simulink library "inp"  (Simulink 8.2)
settings: Solver=ode45  SolverMode=SingleTasking  StartTime=0.0  StopTime=10.0  RelTol=1e-3
undefined symbols (not defined in the file or in its folder): C_TICK_TIME_S, ENUM_FAULTSTATE_FAIL, ENUM_FAULTSTATE_INDETERMINATE, ENUM_FAULTSTATE_PASS, ENUM_KEYSWITCH_ON, FALSE, KINP_ABS1CANCommuOk_flg_ovrdflg, KINP_ABS1CANCommuOk_flg_ovrdval, KINP_ABS1CANPeriodTime_s, KINP_ABS1CANRolCntOk_flg_ovrdflg, KINP_ABS1CANRolCntOk_flg_ovrdval, KINP_ABS2CANChkSumOk_flg_ovrdflg, KINP_ABS2CANChkSumOk_flg_ovrdval, KINP_ABS2CANCommuOk_flg_ovrdflg, KINP_ABS2CANCommuOk_flg_ovrdval, KINP_ABS2CANPeriodTime_s, KINP_ABS2CANRolCntOk_flg_ovrdflg, KINP_ABS2CANRolCntOk_flg_ovrdval, KINP_ABS3CANCommuOk_flg_ovrdflg, KINP_ABS3CANCommuOk_flg_ovrdval, KINP_ABS4CANCommuOk_flg_ovrdflg, KINP_ABS4CANCommuOk_flg_ovrdval, KINP_ABSActive_flg_defval, KINP_ABSActive_flg_invalid, KINP_ABSActive_flg_ovrdflg, KINP_ABSActive_flg_ovrdval, KINP_ABSActive_flg_setvalid, KINP_ABSActive_flg_srvcflg, KINP_ABSActive_flg_srvcval, KINP_ABSCANAlive_flg_ovrdflg, KINP_ABSCANAlive_flg_ovrdval, KINP_ABSCANCommuOk_flg_ovrdflg, KINP_ABSCANCommuOk_flg_ovrdval, KINP_ABSCANValidOk_flg_ovrdflg, KINP_ABSCANValidOk_flg_ovrdval, KINP_ABSFault_flg_defval, KINP_ABSFault_flg_invalid, KINP_ABSFault_flg_ovrdflg, KINP_ABSFault_flg_ovrdval, KINP_ABSFault_flg_setvalid, KINP_ABSFault_flg_srvcflg, KINP_ABSFault_flg_srvcval, KINP_AC1CANCommuOk_flg_ovrdflg, KINP_AC1CANCommuOk_flg_ovrdval, KINP_ACCANAlive_flg_ovrdflg, KINP_ACCANAlive_flg_ovrdval, KINP_ACCANCommuOk_flg_ovrdflg, KINP_ACCANCommuOk_flg_ovrdval, KINP_ACCANValidOk_flg_ovrdflg, KINP_ACCANValidOk_flg_ovrdval, KINP_ACCCancelH_flg_defval, KINP_ACCCancelH_flg_invalid, KINP_ACCCancelH_flg_ovrdflg, KINP_ACCCancelH_flg_ovrdval, KINP_ACCCancelH_flg_setvalid, KINP_ACCCancelH_flg_srvcflg, KINP_ACCCancelH_flg_srvcval, KINP_AccelPedalPosn_pct_defval, KINP_AccelPedalPosn_pct_gain, KINP_AccelPedalPosn_pct_invalid ... (+1866)
libraries: inp x192, hcu_lib x34, simulink x33
(part 3 of 21)

== system inp/INP/ECM_signals ==
  BHAL_outputs : Inport  OutDataTypeStr=Bus: BHAL_outputs
  CANvalid_bus : Inport  Port=2
  AccelPedalPosn : Subsystem
      settings: RTWSystemCode=Inline
  Bus Selector1 : BusSelector  OutputSignals=VHAL_OBDWarnCycAchiv_flg
  Bus Selector{7,10,17,20,22} (5 alike) : BusSelector  OutputSignals=VINP_CANMsgValidECM1_flg
  Bus Selector11 : BusSelector  OutputSignals=VHAL_EngTqFriction_Nm,VHAL_EngTqFrictionInvalid_flg
  Bus Selector{12,24,26..27} (4 alike) : BusSelector  OutputSignals=VINP_CANMsgValidECM9_flg,VINP_CANMsgUpdatedECM9_flg
  Bus Selector13 : BusSelector  OutputSignals=VHAL_EngTqMax_Nm,VHAL_EngTqMaxInvalid_flg
  Bus Selector14 : BusSelector  OutputSignals=VHAL_EMSOpState_enum
  Bus Selector15 : BusSelector  OutputSignals=VHAL_EngTqMin_Nm,VHAL_EngTqMinInvalid_flg
  Bus Selector16 : BusSelector  OutputSignals=VINP_CANMsgValidECM2_flg
  Bus Selector18 : BusSelector  OutputSignals=VHAL_EngTqIndicated_Nm,VHAL_EngTqIndicatedInvalid_flg
  Bus Selector2 : BusSelector  OutputSignals=VINP_CANMsgValidECM2_flg
  Bus Selector21 : BusSelector  OutputSignals=VHAL_EngTqIndicatedCOR_Nm,VHAL_EngTqIndicatedCORInvalid_flg
  Bus Selector23 : BusSelector  OutputSignals=VHAL_EngTqUnCORFault_flg
  Bus Selector25 : BusSelector  OutputSignals=VHAL_EMSHybridOpState_enum
  Bus Selector28 : BusSelector  OutputSignals=VHAL_EngHoodOpen_flg
  Bus Selector3 : BusSelector  OutputSignals=VINP_CANMsgValidECM3_flg
  Bus Selector30 : BusSelector  OutputSignals=VINP_CANMsgValidECM2_flg
  Bus Selector4 : BusSelector  OutputSignals=VHAL_AccelPedalPosn_pct,VHAL_AccelPedalPosnInvalid_flg
  Bus Selector5 : BusSelector  OutputSignals=VINP_CANMsgValidECM3_flg
  Bus Selector6 : BusSelector  OutputSignals=VHAL_EffAccelPedalPosn_pct,VHAL_EffAccelPedalPosnInvalid_flg
  Bus Selector8 : BusSelector  OutputSignals=VHAL_OBDDriveCycAchiv_flg
  Bus Selector9 : BusSelector  OutputSignals=VHAL_EngSpd_erpm,VHAL_EngSpdInvalid_flg
  Constant : Constant  Value=TRUE
  Constant1 : Constant  Value=TRUE
  Data Store Read1 : DataStoreRead  DataStoreName=VDDML_ECM2CANCommuFault_AF_flg
  Data Store Read{10,13,25,27} (4 alike) : DataStoreRead  DataStoreName=VDDML_ECM9CANRolCntFault_AF_flg
  Data Store Read11 : DataStoreRead  DataStoreName=VDDML_CANEngTqIndSigFault_AF_flg
  Data Store Read12 : DataStoreRead  DataStoreName=VDDML_CANAPPSigFault_AF_flg
  Data Store Read{9,14,26,28} (4 alike) : DataStoreRead  DataStoreName=VDDML_ECM9CANCommuFault_AF_flg
  Data Store Read16 : DataStoreRead  DataStoreName=VDDML_CANEngSpdSigFault_AF_flg
  Data Store Read17 : DataStoreRead  DataStoreName=VDDML_ECM3CANCommuFault_AF_flg
  Data Store Read18 : DataStoreRead  DataStoreName=VDDML_CANEngTqFricSigFault_AF_flg
  Data Store Read19 : DataStoreRead  DataStoreName=VDDML_CANEngTqMaxSigFault_AF_flg
  Data Store Read2 : DataStoreRead  DataStoreName=VDDML_ECM2CANCommuFault_AF_flg
  Data Store Read20 : DataStoreRead  DataStoreName=VDDML_CANEngTqMinSigFault_AF_flg
  Data Store Read{5,8,21,23..24} (5 alike) : DataStoreRead  DataStoreName=VDDML_ECM1CANCommuFault_AF_flg
  Data Store Read29 : DataStoreRead  DataStoreName=VDDML_ECM2CANCommuFault_AF_flg
  Data Store Read30 : DataStoreRead  DataStoreName=VDDML_CANEngTqIndCORSigFault_AF_flg
  Data Store Read6 : DataStoreRead  DataStoreName=VDDML_CANEffAPPSigFault_AF_flg
  Data Store Read7 : DataStoreRead  DataStoreName=VDDML_ECM3CANCommuFault_AF_flg
  Data Type Conversion : DataTypeConversion  OutDataTypeStr=boolean
  Data Type Conversion1 : DataTypeConversion  OutDataTypeStr=boolean
  EMSHybridOpState : Subsystem
      settings: RTWSystemCode=Inline
  EMSOpState : Subsystem
      settings: RTWSystemCode=Inline
  EffAccelPedalPosn : Subsystem
      settings: RTWSystemCode=Inline
  EngHoodOpen : Subsystem
      settings: RTWSystemCode=Inline
  EngTqUnCORFault : Subsystem
      settings: RTWSystemCode=Inline
  EngineFrictionTorque : Subsystem
      settings: RTWSystemCode=Inline
  EngineIndicatedTorque : Subsystem
      settings: RTWSystemCode=Inline
  EngineIndicatedTorqueCorrected : Subsystem
      settings: RTWSystemCode=Inline
  EngineSpeed : Subsystem
      settings: RTWSystemCode=Inline
  EngineTorqueMax : Subsystem
      settings: RTWSystemCode=Inline
  EngineTorqueMin : Subsystem
      settings: RTWSystemCode=Inline
  Logical Operator{2..4,10,12,20,23,25} (8 alike) : Logic  Tag=struct('inputs','1','comment',struct('text','','placement',1),'logdata',struct('maxnumvals',inf,'plotchannels',[],'stepsize',0,'downsampling',1,'loggingmode',3,'channels',1),'operator','NOT','output',struct('class','default','type','Bool8','description','','address','','unit','','name','$S_$B'),'date',731430.46749816)  Operator=OR
  Logical Operator{5,8,11,13,15,17..19} (8 alike) : Logic  Tag=struct('inputs','1','comment',struct('text','','placement',1),'logdata',struct('maxnumvals',inf,'plotchannels',[],'stepsize',0,'downsampling',1,'loggingmode',3,'channels',1),'operator','NOT','output',struct('class','default','type','Bool8','description','','address','','unit','','name','$S_$B'),'date',731430.46749816)  Operator=NOT  Inputs=1
  Logical Operator14 : Logic  Tag=struct('inputs','1','comment',struct('text','','placement',1),'logdata',struct('maxnumvals',inf,'plotchannels',[],'stepsize',0,'downsampling',1,'loggingmode',3,'channels',1),'operator','NOT','output',struct('class','default','type','Bool8','description','','address','','unit','','name','$S_$B'),'date',731430.46749816)  Operator=OR  Inputs=3
  Logical Operator16 : Logic  Tag=struct('inputs','1','comment',struct('text','','placement',1),'logdata',struct('maxnumvals',inf,'plotchannels',[],'stepsize',0,'downsampling',1,'loggingmode',3,'channels',1),'operator','NOT','output',struct('class','default','type','Bool8','description','','address','','unit','','name','$S_$B'),'date',731430.46749816)  Operator=OR  Inputs=3
  Logical Operator{21..22,24,26} (4 alike) : Logic  Tag=struct('inputs','1','comment',struct('text','','placement',1),'logdata',struct('maxnumvals',inf,'plotchannels',[],'stepsize',0,'downsampling',1,'loggingmode',3,'channels',1),'operator','NOT','output',struct('class','default','type','Bool8','description','','address','','unit','','name','$S_$B'),'date',731430.46749816)
  Terminator{,1..3} (4 alike) : Terminator
  inputs_bus2 : BusCreator  Inputs=36
  ECM_signal_bus : Outport
  wires:
  CANvalid_bus/1 -> [CANvalid_bus]
  Logical Operator8/1 -> AccelPedalPosn/2(signal_valid_in)
  inputs_bus2/1 -> ECM_signal_bus/1
  Bus Selector4/1 -> AccelPedalPosn/1(signal_in)  "<VHAL_AccelPedalPosn_pct>"
  BHAL_outputs/1 -> [BHAL_outputs]
  [BHAL_outputs] -> Bus Selector4/1
  AccelPedalPosn/2(signal_valid_out) -> inputs_bus2/2  "VINP_AccelPedalPosnValid_flg_InHAL"
  AccelPedalPosn/1(signal_out) -> inputs_bus2/1  "VINP_AccelPedalPosn_pct_InHAL"
  Bus Selector4/2 -> Logical Operator8/1  "<VHAL_AccelPedalPosnInvalid_flg>"
  Data Store Read12/1 -> Logical Operator2/1
  [CANvalid_bus] -> Bus Selector3/1
  Bus Selector3/1 -> AccelPedalPosn/3(msg_valid)  "<VINP_CANMsgValidECM3_flg>"
  Logical Operator2/1 -> AccelPedalPosn/4(Active_fault_flg)
  Data Store Read17/1 -> Logical Operator2/2
  AccelPedalPosn/3(raw_signal_valid_out) -> inputs_bus2/3  "VINP_AccelPedalPosnSigOk_flg_InHAL"
  Logical Operator5/1 -> EffAccelPedalPosn/2(signal_valid_in)
  Bus Selector6/1 -> EffAccelPedalPosn/1(signal_in)  "<VHAL_EffAccelPedalPosn_pct>"
  [BHAL_outputs] -> Bus Selector6/1
  Bus Selector6/2 -> Logical Operator5/1  "<VHAL_EffAccelPedalPosnInvalid_flg>"
  Data Store Read6/1 -> Logical Operator4/1
  [CANvalid_bus] -> Bus Selector5/1
  Bus Selector5/1 -> EffAccelPedalPosn/3(msg_valid)  "<VINP_CANMsgValidECM3_flg>"
  Logical Operator4/1 -> EffAccelPedalPosn/4(Active_fault_flg)
  Data Store Read7/1 -> Logical Operator4/2
  EffAccelPedalPosn/1(signal_out) -> inputs_bus2/4  "VINP_EffAccelPedalPosn_pct_InHAL"
  EffAccelPedalPosn/2(signal_valid_out) -> inputs_bus2/5  "VINP_EffAccelPedalPosnValid_flg_InHAL"
  EffAccelPedalPosn/3(raw_signal_valid_out) -> inputs_bus2/6  "VINP_EffAccelPedalPosnSigOk_flg_InHAL"
  Logical Operator11/1 -> EngineSpeed/2(signal_valid_in)
  Bus Selector9/1 -> EngineSpeed/1(signal_in)  "<VHAL_EngSpd_erpm>"
  [BHAL_outputs] -> Bus Selector9/1
  Bus Selector9/2 -> Logical Operator11/1  "<VHAL_EngSpdInvalid_flg>"
  Data Store Read16/1 -> Logical Operator10/1
  [CANvalid_bus] -> Bus Selector7/1
  Bus Selector7/1 -> EngineSpeed/3(msg_valid)  "<VINP_CANMsgValidECM1_flg>"
  Logical Operator10/1 -> EngineSpeed/4(Active_fault_flg)
  Data Store Read8/1 -> Logical Operator10/2
  EngineSpeed/1(signal_out) -> inputs_bus2/7  "VINP_EngSpd_erpm_InHAL"
  EngineSpeed/2(signal_valid_out) -> inputs_bus2/8  "VINP_EngSpdValid_flg_InHAL"
  EngineSpeed/3(raw_signal_valid_out) -> inputs_bus2/9  "VINP_EngSpdSigOk_flg_InHAL"
  Logical Operator13/1 -> EngineFrictionTorque/2(signal_valid_in)
  Bus Selector11/1 -> EngineFrictionTorque/1(signal_in)  "<VHAL_EngTqFriction_Nm>"
  [BHAL_outputs] -> Bus Selector11/1
  Bus Selector11/2 -> Logical Operator13/1  "<VHAL_EngTqFrictionInvalid_flg>"
  Data Store Read18/1 -> Logical Operator12/1
  [CANvalid_bus] -> Bus Selector10/1
  Bus Selector10/1 -> EngineFrictionTorque/3(msg_valid)  "<VINP_CANMsgValidECM1_flg>"
  Logical Operator12/1 -> EngineFrictionTorque/4(Active_fault_flg)
  Data Store Read5/1 -> Logical Operator12/2
  EngineFrictionTorque/1(signal_out) -> inputs_bus2/10  "VINP_EngTqFriction_Nm_InHAL"
  EngineFrictionTorque/2(signal_valid_out) -> inputs_bus2/11  "VINP_EngTqFrictionValid_flg_InHAL"
  EngineFrictionTorque/3(raw_signal_valid_out) -> inputs_bus2/12  "VINP_EngTqFrictionSigOk_flg_InHAL"
  Logical Operator15/1 -> EngineTorqueMax/2(signal_valid_in)
  Bus Selector13/1 -> EngineTorqueMax/1(signal_in)  "<VHAL_EngTqMax_Nm>"
  [BHAL_outputs] -> Bus Selector13/1
  Bus Selector13/2 -> Logical Operator15/1  "<VHAL_EngTqMaxInvalid_flg>"
  [CANvalid_bus] -> Bus Selector12/1
  Bus Selector12/1 -> Logical Operator21/1  "<VINP_CANMsgValidECM9_flg>"
  Logical Operator14/1 -> EngineTorqueMax/4(Active_fault_flg)
  EngineTorqueMax/1(signal_out) -> inputs_bus2/21  "VINP_EngTqMax_Nm_InHAL"
  EngineTorqueMax/2(signal_valid_out) -> inputs_bus2/22  "VINP_EngTqMaxValid_flg_InHAL"
  EngineTorqueMax/3(raw_signal_valid_out) -> inputs_bus2/23  "VINP_EngTqMaxSigOk_flg_InHAL"
  Data Store Read19/1 -> Logical Operator14/1
  Data Store Read9/1 -> Logical Operator14/2
  Data Store Read10/1 -> Logical Operator14/3
  Logical Operator17/1 -> EngineTorqueMin/2(signal_valid_in)
  Bus Selector15/1 -> EngineTorqueMin/1(signal_in)  "<VHAL_EngTqMin_Nm>"
  [BHAL_outputs] -> Bus Selector15/1
  Bus Selector15/2 -> Logical Operator17/1  "<VHAL_EngTqMinInvalid_flg>"
  [CANvalid_bus] -> Bus Selector24/1
  Logical Operator16/1 -> EngineTorqueMin/4(Active_fault_flg)
  EngineTorqueMin/1(signal_out) -> inputs_bus2/24  "VINP_EngTqMin_Nm_InHAL"
  EngineTorqueMin/2(signal_valid_out) -> inputs_bus2/25  "VINP_EngTqMinValid_flg_InHAL"
  EngineTorqueMin/3(raw_signal_valid_out) -> inputs_bus2/26  "VINP_EngTqMinSigOk_flg_InHAL"
  Data Store Read20/1 -> Logical Operator16/1
  Data Store Read14/1 -> Logical Operator16/2
  Data Store Read13/1 -> Logical Operator16/3
  Logical Operator18/1 -> EngineIndicatedTorque/2(signal_valid_in)
  Bus Selector18/1 -> EngineIndicatedTorque/1(signal_in)  "<VHAL_EngTqIndicated_Nm>"
  [BHAL_outputs] -> Bus Selector18/1
  Bus Selector18/2 -> Logical Operator18/1  "<VHAL_EngTqIndicatedInvalid_flg>"
  Data Store Read11/1 -> Logical Operator3/1
  [CANvalid_bus] -> Bus Selector17/1
  Bus Selector17/1 -> EngineIndicatedTorque/3(msg_valid)  "<VINP_CANMsgValidECM1_flg>"
  Logical Operator3/1 -> EngineIndicatedTorque/4(Active_fault_flg)
  Data Store Read21/1 -> Logical Operator3/2
  EngineIndicatedTorque/1(signal_out) -> inputs_bus2/13  "VINP_EngTqIndicated_Nm_InHAL"
  EngineIndicatedTorque/2(signal_valid_out) -> inputs_bus2/14  "VINP_EngTqIndicatedValid_flg_InHAL"
  EngineIndicatedTorque/3(raw_signal_valid_out) -> inputs_bus2/15  "VINP_EngTqIndicatedSigOk_flg_InHAL"
  Logical Operator19/1 -> EngineIndicatedTorqueCorrected/2(signal_valid_in)
  Bus Selector21/1 -> EngineIndicatedTorqueCorrected/1(signal_in)  "<VHAL_EngTqIndicatedCOR_Nm>"
  [BHAL_outputs] -> Bus Selector21/1
  Bus Selector21/2 -> Logical Operator19/1  "<VHAL_EngTqIndicatedCORInvalid_flg>"
  Data Store Read30/1 -> Logical Operator20/1
  [CANvalid_bus] -> Bus Selector20/1
  Bus Selector20/1 -> EngineIndicatedTorqueCorrected/3(msg_valid)  "<VINP_CANMsgValidECM1_flg>"
  Logical Operator20/1 -> EngineIndicatedTorqueCorrected/4(Active_fault_flg)
  Data Store Read23/1 -> Logical Operator20/2
  EngineIndicatedTorqueCorrected/1(signal_out) -> inputs_bus2/16  "VINP_EngTqIndicatedCOR_Nm_InHAL"
  EngineIndicatedTorqueCorrected/2(signal_valid_out) -> inputs_bus2/17  "VINP_EngTqIndicatedCORValid_flg_InHAL"
  EngineIndicatedTorqueCorrected/3(raw_signal_valid_out) -> inputs_bus2/18  "VINP_EngTqIndicatedCORSigOk_flg_InHAL"
  Bus Selector23/1 -> EngTqUnCORFault/1(signal_in)  "<VHAL_EngTqUnCORFault_flg>"
  [BHAL_outputs] -> Bus Selector23/1
  Bus Selector22/1 -> EngTqUnCORFault/2(msg_valid)  "<VINP_CANMsgValidECM1_flg>"
  [CANvalid_bus] -> Bus Selector22/1
  Data Store Read24/1 -> EngTqUnCORFault/3(Active_fault_flg)
  EngTqUnCORFault/1(signal_out) -> inputs_bus2/19  "VINP_EngTqUnCORFault_flg_InHAL"
  EngTqUnCORFault/2(signal_valid_out) -> inputs_bus2/20  "VINP_EngTqUnCORFaultValid_flg_InHAL"
  Logical Operator21/1 -> EngineTorqueMax/3(msg_valid)
  Bus Selector12/2 -> Logical Operator21/2  "<VINP_CANMsgUpdatedECM9_flg>"
  Bus Selector24/1 -> Logical Operator22/1  "<VINP_CANMsgValidECM9_flg>"
  Bus Selector24/2 -> Logical Operator22/2  "<VINP_CANMsgUpdatedECM9_flg>"
  Logical Operator22/1 -> EngineTorqueMin/3(msg_valid)
  Bus Selector25/1 -> EMSHybridOpState/1(signal_in)  "<VHAL_EMSHybridOpState_enum>"
  [BHAL_outputs] -> Bus Selector25/1
  EMSHybridOpState/1(signal_out) -> inputs_bus2/27  "VINP_EMSHybridOpState_enum_InHAL"
  EMSHybridOpState/2(signal_valid_out) -> inputs_bus2/28  "VINP_EMSHybridOpStateValid_flg_InHAL"
  Logical Operator23/1 -> EMSHybridOpState/3(Active_fault_flg)
  Data Store Read26/1 -> Logical Operator23/1
  Data Store Read25/1 -> Logical Operator23/2
  [CANvalid_bus] -> Bus Selector26/1
  Bus Selector26/1 -> Logical Operator24/1  "<VINP_CANMsgValidECM9_flg>"
  Bus Selector26/2 -> Logical Operator24/2  "<VINP_CANMsgUpdatedECM9_flg>"
  Logical Operator24/1 -> EMSHybridOpState/2(msg_valid)
  Bus Selector14/1 -> EMSOpState/1(signal_in)  "<VHAL_EMSOpState_enum>"
  [BHAL_outputs] -> Bus Selector14/1
  EMSOpState/1(signal_out) -> inputs_bus2/29  "VINP_EMSOpState_enum_InHAL"
  EMSOpState/2(signal_valid_out) -> inputs_bus2/30  "VINP_EMSOpStateValid_flg_InHAL"
  Logical Operator25/1 -> EMSOpState/3(Active_fault_flg)
  Data Store Read28/1 -> Logical Operator25/1
  Data Store Read27/1 -> Logical Operator25/2
  [CANvalid_bus] -> Bus Selector27/1
  Bus Selector27/1 -> Logical Operator26/1  "<VINP_CANMsgValidECM9_flg>"
  Bus Selector27/2 -> Logical Operator26/2  "<VINP_CANMsgUpdatedECM9_flg>"
  Logical Operator26/1 -> EMSOpState/2(msg_valid)
  Bus Selector28/1 -> EngHoodOpen/1(signal_in)  "<VHAL_EngHoodOpen_flg>"
  [BHAL_outputs] -> Bus Selector28/1
  EngHoodOpen/1(signal_out) -> inputs_bus2/31  "VINP_EngHoodOpen_flg_InHAL"
  EngHoodOpen/2(signal_valid_out) -> inputs_bus2/32  "VINP_EngHoodOpenValid_flg_InHAL"
  Bus Selector30/1 -> EngHoodOpen/2(msg_valid)  "<VINP_CANMsgValidECM2_flg>"
  [CANvalid_bus] -> Bus Selector30/1
  Data Store Read29/1 -> EngHoodOpen/3(Active_fault_flg)
  Bus Selector1/1 -> Data Type Conversion/1  "<VHAL_OBDWarnCycAchiv_flg>"
  [BHAL_outputs] -> Bus Selector1/1
  Data Type Conversion/1 -> inputs_bus2/33  "VINP_OBDWarnCycAchiv_flg_InHAL"
  Constant/1 -> inputs_bus2/34  "VINP_OBDWarnCycAchivValid_flg_InHAL"
  Bus Selector2/1 -> Terminator/1  "<VINP_CANMsgValidECM2_flg>"
  [CANvalid_bus] -> Bus Selector2/1
  Data Store Read1/1 -> Terminator1/1
  Bus Selector8/1 -> Data Type Conversion1/1  "<VHAL_OBDDriveCycAchiv_flg>"
  [BHAL_outputs] -> Bus Selector8/1
  Data Type Conversion1/1 -> inputs_bus2/35  "VINP_OBDDriveCycAchiv_flg_InHAL"
  Constant1/1 -> inputs_bus2/36  "VINP_OBDDriveCycAchivValid_flg_InHAL"
  Bus Selector16/1 -> Terminator2/1  "<VINP_CANMsgValidECM2_flg>"
  [CANvalid_bus] -> Bus Selector16/1
  Data Store Read2/1 -> Terminator3/1

== system inp/INP/EPB_signals ==
  BHAL_outputs : Inport  OutDataTypeStr=Bus: BHAL_outputs
  CANvalid_bus : Inport  Port=2
  Bus Selector8 : BusSelector  OutputSignals=VHAL_ParkBrakeBindSta_enum,VHAL_ParkBrakeBindStaInvalid_flg
  Bus Selector9 : BusSelector  OutputSignals=VINP_CANMsgValidEPB1_flg
  Data Store Read13 : DataStoreRead  DataStoreName=VDDML_EPB1CANCommuFault_AF_flg
  Data Store Read14 : DataStoreRead  DataStoreName=VDDML_CANParkBrakBindStaSigFault_AF_flg
  Logical Operator14 : Logic  Tag=struct('inputs','1','comment',struct('text','','placement',1),'logdata',struct('maxnumvals',inf,'plotchannels',[],'stepsize',0,'downsampling',1,'loggingmode',3,'channels',1),'operator','NOT','output',struct('class','default','type','Bool8','description','','address','','unit','','name','$S_$B'),'date',731430.46749816)  Operator=NOT
  Logical Operator6 : Logic  Tag=struct('inputs','1','comment',struct('text','','placement',1),'logdata',struct('maxnumvals',inf,'plotchannels',[],'stepsize',0,'downsampling',1,'loggingmode',3,'channels',1),'operator','NOT','output',struct('class','default','type','Bool8','description','','address','','unit','','name','$S_$B'),'date',731430.46749816)  Operator=OR
  ParkBrakeBindSta : Subsystem
      settings: RTWSystemCode=Inline
  inputs_bus2 : BusCreator  Inputs=3
  EPB_signal_bus : Outport
  wires:
  Logical Operator6/1 -> ParkBrakeBindSta/4(Active_fault_flg)
  Bus Selector9/1 -> ParkBrakeBindSta/3(msg_valid)  "<VINP_CANMsgValidEPB1_flg>"
  [CANvalid_bus] -> Bus Selector9/1
  ParkBrakeBindSta/3(raw_signal_valid_out) -> inputs_bus2/3  "VINP_ParkBrakeBindStaSigOk_flg_InHAL"
  ParkBrakeBindSta/2(signal_valid_out) -> inputs_bus2/2  "VINP_ParkBrakeBindStaValid_flg_InHAL"
  ParkBrakeBindSta/1(signal_out) -> inputs_bus2/1  "VINP_ParkBrakeBindSta_enum_InHAL"
  Data Store Read13/1 -> Logical Operator6/2
  Data Store Read14/1 -> Logical Operator6/1
  Logical Operator14/1 -> ParkBrakeBindSta/2(signal_valid_in)
  Bus Selector8/2 -> Logical Operator14/1  "<VHAL_ParkBrakeBindStaInvalid_flg>"
  Bus Selector8/1 -> ParkBrakeBindSta/1(signal_in)  "<VHAL_ParkBrakeBindSta_enum>"
  [BHAL_outputs] -> Bus Selector8/1
  CANvalid_bus/1 -> [CANvalid_bus]
  BHAL_outputs/1 -> [BHAL_outputs]
  inputs_bus2/1 -> EPB_signal_bus/1

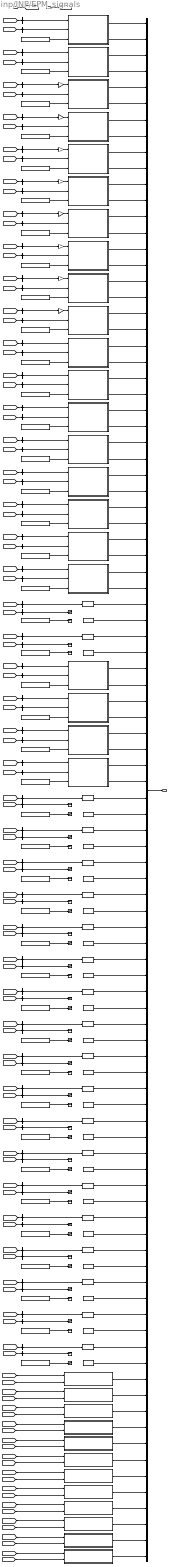
== system inp/INP/EPM_signals ==
  BHAL_outputs : Inport  OutDataTypeStr=Bus: BHAL_outputs
  CANvalid_bus : Inport  Port=2
  Bus Selector1 : BusSelector  OutputSignals=VHAL_LaneQualityR_enum
  Bus Selector{2,10,14,16,21,31} (6 alike) : BusSelector  OutputSignals=VINP_CANMsgValidEPM_flg.VINP_CANMsgValidEPM3_flg
  Bus Selector11 : BusSelector  OutputSignals=VHAL_LaneC0R_m
  Bus Selector{12,27,35,39,43,49} (6 alike) : BusSelector  OutputSignals=VINP_CANMsgValidEPM_flg.VINP_CANMsgValidEPM4_flg
  Bus Selector13 : BusSelector  OutputSignals=VHAL_LaneC1R_rad
  Bus Selector15 : BusSelector  OutputSignals=VHAL_LaneC2R_rat
  Bus Selector17 : BusSelector  OutputSignals=VHAL_LaneC3R_rat
  Bus Selector{5,7..8,18,23,29} (6 alike) : BusSelector  OutputSignals=VINP_CANMsgValidEPM_flg.VINP_CANMsgValidEPM1_flg
  Bus Selector19 : BusSelector  OutputSignals=VHAL_LaneQualityL_enum
  Bus Selector20 : BusSelector  OutputSignals=VHAL_LaneTypeL_enum
  Bus Selector22 : BusSelector  OutputSignals=VHAL_LaneTypeR_enum
  Bus Selector24 : BusSelector  OutputSignals=VHAL_LaneC0L_m
  Bus Selector{3,25,33,37,41,46} (6 alike) : BusSelector  OutputSignals=VINP_CANMsgValidEPM_flg.VINP_CANMsgValidEPM2_flg
  Bus Selector26 : BusSelector  OutputSignals=VHAL_LaneMrkClrL_enum
  Bus Selector28 : BusSelector  OutputSignals=VHAL_LaneMrkClrR_enum
  Bus Selector30 : BusSelector  OutputSignals=VHAL_LaneMrkWidL_m
  Bus Selector32 : BusSelector  OutputSignals=VHAL_LaneMrkWidR_m
  Bus Selector34 : BusSelector  OutputSignals=VHAL_ViewRangeStartL_m
  Bus Selector36 : BusSelector  OutputSignals=VHAL_ViewRangeStartR_m
  Bus Selector38 : BusSelector  OutputSignals=VHAL_TLCL_s
  Bus Selector4 : BusSelector  OutputSignals=VHAL_LaneC1L_rad
  Bus Selector40 : BusSelector  OutputSignals=VHAL_TLCR_s
  Bus Selector42 : BusSelector  OutputSignals=VHAL_LaneCrosL_flg
  Bus Selector44 : BusSelector  OutputSignals=VHAL_LaneCrosR_flg
  Bus Selector{45,47,53,55,57,59,61,63,65,67,69,71,73,75,77,79,81,83} (18 alike) : BusSelector  OutputSignals=VINP_CANMsgValidEPM_flg.VINP_CANMsgValidEPM5_flg
  Bus Selector48 : BusSelector  OutputSignals=VHAL_ViewRangeEndL_m
  Bus Selector50 : BusSelector  OutputSignals=VHAL_ViewRangeEndR_m
  Bus Selector51 : BusSelector  OutputSignals=VHAL_CamGoDecision_enum
  Bus Selector52 : BusSelector  OutputSignals=VHAL_CamNofObjects_num
  Bus Selector54 : BusSelector  OutputSignals=VHAL_CloseCarDetected_flg
  Bus Selector56 : BusSelector  OutputSignals=VHAL_RightCarCutIn_flg
  Bus Selector58 : BusSelector  OutputSignals=VHAL_LeftCarCutIn_flg
  Bus Selector6 : BusSelector  OutputSignals=VHAL_LaneC2L_rat
  Bus Selector60 : BusSelector  OutputSignals=VHAL_CamFSImpairedVD_flg
  Bus Selector62 : BusSelector  OutputSignals=VHAL_CamFSLowSun_flg
  Bus Selector64 : BusSelector  OutputSignals=VHAL_CamFSBlurImage_flg
  Bus Selector66 : BusSelector  OutputSignals=VHAL_CamFSSmearImage_flg
  Bus Selector68 : BusSelector  OutputSignals=VHAL_CamFSPartialBlockage_flg
  Bus Selector70 : BusSelector  OutputSignals=VHAL_CamFSFullBlockage_flg
  Bus Selector72 : BusSelector  OutputSignals=VHAL_CamFSSplashes_flg
  Bus Selector74 : BusSelector  OutputSignals=VHAL_CamFSOutofCal_flg
  Bus Selector76 : BusSelector  OutputSignals=VHAL_CamFSCalMisalign_flg
  Bus Selector78 : BusSelector  OutputSignals=VHAL_CamFSSmearedspots_flg
  Bus Selector80 : BusSelector  OutputSignals=VHAL_CamFSSpotHalosFrozen_flg
  Bus Selector82 : BusSelector  OutputSignals=VHAL_CamFSFoggySpots_flg
  Bus Selector84 : BusSelector  OutputSignals=VHAL_CamFSSunRay_flg
  Bus Selector9 : BusSelector  OutputSignals=VHAL_LaneC3L_rat
  Cam_Left_Next_Lane : Subsystem
  Cam_Obstacle_1 : Subsystem
  Cam_Obstacle_10 : Subsystem
  Cam_Obstacle_2 : Subsystem
  Cam_Obstacle_3 : Subsystem
  Cam_Obstacle_4 : Subsystem
  Cam_Obstacle_5 : Subsystem
  Cam_Obstacle_6 : Subsystem
  Cam_Obstacle_7 : Subsystem
  Cam_Obstacle_8 : Subsystem
  Cam_Obstacle_9 : Subsystem
  Cam_Right_Next_Lane : Subsystem
  Constant{1..20} (20 alike) : Constant  Value=TRUE
  Data Store Read{1,7,9..10,12,16} (6 alike) : DataStoreRead  DataStoreName=VDDML_EPM3CANCommuFault_AF_flg
  Data Store Read{2,4..6,11,15} (6 alike) : DataStoreRead  DataStoreName=VDDML_EPM1CANCommuFault_AF_flg
  Data Store Read{3,13,17,19,21,26} (6 alike) : DataStoreRead  DataStoreName=VDDML_EPM2CANCommuFault_AF_flg
  Data Store Read{8,14,18,20,22,25} (6 alike) : DataStoreRead  DataStoreName=VDDML_EPM4CANCommuFault_AF_flg
  Data Store Read{23..24,27..42} (18 alike) : DataStoreRead  DataStoreName=VDDML_EPM5CANCommuFault_AF_flg
  Data Type Conversion1 : DataTypeConversion  OutDataTypeStr=single
  Data Type Conversion{5..20} (16 alike) : DataTypeConversion  OutDataTypeStr=boolean
  Data Type Conversion2 : DataTypeConversion  OutDataTypeStr=single
  Data Type Conversion3 : DataTypeConversion  OutDataTypeStr=uint8
  Data Type Conversion4 : DataTypeConversion  OutDataTypeStr=uint8
  Gain{,1..7} (8 alike) : Gain  Gain=-1
  LaneC0Left : Subsystem
      settings: RTWSystemCode=Inline
  LaneC0Right : Subsystem
      settings: RTWSystemCode=Inline
  LaneC1Left : Subsystem
      settings: RTWSystemCode=Inline
  LaneC1Right : Subsystem
      settings: RTWSystemCode=Inline
  LaneC2Left : Subsystem
      settings: RTWSystemCode=Inline
  LaneC2Right : Subsystem
      settings: RTWSystemCode=Inline
  LaneC3Left : Subsystem
      settings: RTWSystemCode=Inline
  LaneC3Right : Subsystem
      settings: RTWSystemCode=Inline
  LaneCrossingLeft : Subsystem
      settings: RTWSystemCode=Inline
  LaneCrossingRight : Subsystem
      settings: RTWSystemCode=Inline
  LaneMarkColorLeft : Subsystem
      settings: RTWSystemCode=Inline
  LaneMarkColorRight : Subsystem
      settings: RTWSystemCode=Inline
  LaneMarkWidthLeft : Subsystem
      settings: RTWSystemCode=Inline
  LaneMarkWidthRight : Subsystem
      settings: RTWSystemCode=Inline
  LaneQualityLeft : Subsystem
      settings: RTWSystemCode=Inline
  LaneQualityRight : Subsystem
      settings: RTWSystemCode=Inline
  LaneTypeLeft : Subsystem
      settings: RTWSystemCode=Inline
  LaneTypeRight : Subsystem
      settings: RTWSystemCode=Inline
  Terminator{1..40} (40 alike) : Terminator
  TimeToLaneCrossingLeft : Subsystem
      settings: RTWSystemCode=Inline
  TimeToLaneCrossingRight : Subsystem
      settings: RTWSystemCode=Inline
  ViewRangeStartL : Subsystem
      settings: RTWSystemCode=Inline
  ViewRangeStartR : Subsystem
      settings: RTWSystemCode=Inline
  inputs_bus2 : BusCreator  Inputs=96
  EPM_signal_bus : Outport
  wires:
  inputs_bus2/1 -> EPM_signal_bus/1
  BHAL_outputs/1 -> [BHAL_outputs]
  Bus Selector19/1 -> LaneQualityLeft/1(signal_in)  "<VHAL_LaneQualityL_enum>"
  [BHAL_outputs] -> Bus Selector19/1
  LaneQualityLeft/1(signal_out) -> inputs_bus2/1  "VINP_LaneQualityL_enum_InHAL"
  LaneQualityLeft/2(signal_valid_out) -> inputs_bus2/2  "VINP_LaneQualityLValid_flg_InHAL"
  Bus Selector23/1 -> LaneC0Left/2(msg_valid)  "<VINP_CANMsgValidEPM1_flg>"
  [CANvalid_bus] -> Bus Selector23/1
  LaneC0Left/1(signal_out) -> inputs_bus2/5  "VINP_LaneC0L_m_InHAL"
  [BHAL_outputs] -> Bus Selector24/1
  Bus Selector24/1 -> Gain/1  "<VHAL_LaneC0L_m>"
  LaneC0Left/2(signal_valid_out) -> inputs_bus2/6  "VINP_LaneC0LValid_flg_InHAL"
  Bus Selector2/1 -> LaneQualityRight/2(msg_valid)  "<VINP_CANMsgValidEPM3_flg>"
  [CANvalid_bus] -> Bus Selector2/1
  Bus Selector1/1 -> LaneQualityRight/1(signal_in)  "<VHAL_LaneQualityR_enum>"
  [BHAL_outputs] -> Bus Selector1/1
  LaneQualityRight/1(signal_out) -> inputs_bus2/3  "VINP_LaneQualityR_enum_InHAL"
  LaneQualityRight/2(signal_valid_out) -> inputs_bus2/4  "VINP_LaneQualityRValid_flg_InHAL"
  Bus Selector3/1 -> LaneC1Left/2(msg_valid)  "<VINP_CANMsgValidEPM2_flg>"
  [CANvalid_bus] -> Bus Selector3/1
  LaneC1Left/1(signal_out) -> inputs_bus2/7  "VINP_LaneC1L_rad_InHAL"
  [BHAL_outputs] -> Bus Selector4/1
  Bus Selector4/1 -> Gain1/1  "<VHAL_LaneC1L_rad>"
  LaneC1Left/2(signal_valid_out) -> inputs_bus2/8  "VINP_LaneC1LValid_flg_InHAL"
  Bus Selector5/1 -> LaneC2Left/2(msg_valid)  "<VINP_CANMsgValidEPM1_flg>"
  [CANvalid_bus] -> Bus Selector5/1
  LaneC2Left/1(signal_out) -> inputs_bus2/9  "VINP_LaneC2L_rat_InHAL"
  [BHAL_outputs] -> Bus Selector6/1
  Bus Selector6/1 -> Gain2/1  "<VHAL_LaneC2L_rat>"
  LaneC2Left/2(signal_valid_out) -> inputs_bus2/10  "VINP_LaneC2LValid_flg_InHAL"
  Bus Selector8/1 -> LaneC3Left/2(msg_valid)  "<VINP_CANMsgValidEPM1_flg>"
  [CANvalid_bus] -> Bus Selector8/1
  LaneC3Left/1(signal_out) -> inputs_bus2/11  "VINP_LaneC3L_rat_InHAL"
  [BHAL_outputs] -> Bus Selector9/1
  Bus Selector9/1 -> Gain3/1  "<VHAL_LaneC3L_rat>"
  LaneC3Left/2(signal_valid_out) -> inputs_bus2/12  "VINP_LaneC3LValid_flg_InHAL"
  Bus Selector10/1 -> LaneC0Right/2(msg_valid)  "<VINP_CANMsgValidEPM3_flg>"
  [CANvalid_bus] -> Bus Selector10/1
  LaneC0Right/1(signal_out) -> inputs_bus2/13  "VINP_LaneC0R_m_InHAL"
  [BHAL_outputs] -> Bus Selector11/1
  Bus Selector11/1 -> Gain4/1  "<VHAL_LaneC0R_m>"
  LaneC0Right/2(signal_valid_out) -> inputs_bus2/14  "VINP_LaneC0RValid_flg_InHAL"
  Bus Selector12/1 -> LaneC1Right/2(msg_valid)  "<VINP_CANMsgValidEPM4_flg>"
  [CANvalid_bus] -> Bus Selector12/1
  LaneC1Right/1(signal_out) -> inputs_bus2/15  "VINP_LaneC1R_rad_InHAL"
  [BHAL_outputs] -> Bus Selector13/1
  Bus Selector13/1 -> Gain5/1  "<VHAL_LaneC1R_rad>"
  LaneC1Right/2(signal_valid_out) -> inputs_bus2/16  "VINP_LaneC1RValid_flg_InHAL"
  Bus Selector14/1 -> LaneC2Right/2(msg_valid)  "<VINP_CANMsgValidEPM3_flg>"
  [CANvalid_bus] -> Bus Selector14/1
  LaneC2Right/1(signal_out) -> inputs_bus2/17  "VINP_LaneC2R_rat_InHAL"
  [BHAL_outputs] -> Bus Selector15/1
  Bus Selector15/1 -> Gain6/1  "<VHAL_LaneC2R_rat>"
  LaneC2Right/2(signal_valid_out) -> inputs_bus2/18  "VINP_LaneC2RValid_flg_InHAL"
  Bus Selector16/1 -> LaneC3Right/2(msg_valid)  "<VINP_CANMsgValidEPM3_flg>"
  [CANvalid_bus] -> Bus Selector16/1
  LaneC3Right/1(signal_out) -> inputs_bus2/19  "VINP_LaneC3R_rat_InHAL"
  [BHAL_outputs] -> Bus Selector17/1
  Bus Selector17/1 -> Gain7/1  "<VHAL_LaneC3R_rat>"
  LaneC3Right/2(signal_valid_out) -> inputs_bus2/20  "VINP_LaneC3RValid_flg_InHAL"
  Bus Selector7/1 -> LaneQualityLeft/2(msg_valid)  "<VINP_CANMsgValidEPM1_flg>"
  [CANvalid_bus] -> Bus Selector7/1
  CANvalid_bus/1 -> [CANvalid_bus]
  Data Store Read4/1 -> LaneQualityLeft/3(Active_fault_flg)
  Data Store Read1/1 -> LaneQualityRight/3(Active_fault_flg)
  Data Store Read2/1 -> LaneC0Left/3(Active_fault_flg)
  Data Store Read3/1 -> LaneC1Left/3(Active_fault_flg)
  Data Store Read5/1 -> LaneC2Left/3(Active_fault_flg)
  Data Store Read6/1 -> LaneC3Left/3(Active_fault_flg)
  Data Store Read7/1 -> LaneC0Right/3(Active_fault_flg)
  Data Store Read8/1 -> LaneC1Right/3(Active_fault_flg)
  Data Store Read9/1 -> LaneC2Right/3(Active_fault_flg)
  Data Store Read10/1 -> LaneC3Right/3(Active_fault_flg)
  Bus Selector18/1 -> LaneTypeLeft/2(msg_valid)  "<VINP_CANMsgValidEPM1_flg>"
  [CANvalid_bus] -> Bus Selector18/1
  [BHAL_outputs] -> Bus Selector20/1
  Bus Selector20/1 -> LaneTypeLeft/1(signal_in)  "<VHAL_LaneTypeL_enum>"
  Data Store Read11/1 -> LaneTypeLeft/3(Active_fault_flg)
  Bus Selector21/1 -> LaneTypeRight/2(msg_valid)  "<VINP_CANMsgValidEPM3_flg>"
  [CANvalid_bus] -> Bus Selector21/1
  [BHAL_outputs] -> Bus Selector22/1
  Bus Selector22/1 -> LaneTypeRight/1(signal_in)  "<VHAL_LaneTypeR_enum>"
  Data Store Read12/1 -> LaneTypeRight/3(Active_fault_flg)
  Bus Selector25/1 -> LaneMarkColorLeft/2(msg_valid)  "<VINP_CANMsgValidEPM2_flg>"
  [CANvalid_bus] -> Bus Selector25/1
  [BHAL_outputs] -> Bus Selector26/1
  Bus Selector26/1 -> LaneMarkColorLeft/1(signal_in)  "<VHAL_LaneMrkClrL_enum>"
  Data Store Read13/1 -> LaneMarkColorLeft/3(Active_fault_flg)
  Bus Selector27/1 -> LaneMarkColorRight/2(msg_valid)  "<VINP_CANMsgValidEPM4_flg>"
  [CANvalid_bus] -> Bus Selector27/1
  [BHAL_outputs] -> Bus Selector28/1
  Bus Selector28/1 -> LaneMarkColorRight/1(signal_in)  "<VHAL_LaneMrkClrR_enum>"
  Data Store Read14/1 -> LaneMarkColorRight/3(Active_fault_flg)
  Bus Selector29/1 -> LaneMarkWidthLeft/2(msg_valid)  "<VINP_CANMsgValidEPM1_flg>"
  [CANvalid_bus] -> Bus Selector29/1
  [BHAL_outputs] -> Bus Selector30/1
  Bus Selector30/1 -> LaneMarkWidthLeft/1(signal_in)  "<VHAL_LaneMrkWidL_m>"
  Data Store Read15/1 -> LaneMarkWidthLeft/3(Active_fault_flg)
  Bus Selector31/1 -> LaneMarkWidthRight/2(msg_valid)  "<VINP_CANMsgValidEPM3_flg>"
  [CANvalid_bus] -> Bus Selector31/1
  [BHAL_outputs] -> Bus Selector32/1
  Bus Selector32/1 -> LaneMarkWidthRight/1(signal_in)  "<VHAL_LaneMrkWidR_m>"
  Data Store Read16/1 -> LaneMarkWidthRight/3(Active_fault_flg)
  Bus Selector33/1 -> ViewRangeStartL/2(msg_valid)  "<VINP_CANMsgValidEPM2_flg>"
  [CANvalid_bus] -> Bus Selector33/1
  [BHAL_outputs] -> Bus Selector34/1
  Bus Selector34/1 -> ViewRangeStartL/1(signal_in)  "<VHAL_ViewRangeStartL_m>"
  Data Store Read17/1 -> ViewRangeStartL/3(Active_fault_flg)
  Bus Selector35/1 -> ViewRangeStartR/2(msg_valid)  "<VINP_CANMsgValidEPM4_flg>"
  [CANvalid_bus] -> Bus Selector35/1
  [BHAL_outputs] -> Bus Selector36/1
  Bus Selector36/1 -> ViewRangeStartR/1(signal_in)  "<VHAL_ViewRangeStartR_m>"
  Data Store Read18/1 -> ViewRangeStartR/3(Active_fault_flg)
  Bus Selector37/1 -> TimeToLaneCrossingLeft/2(msg_valid)  "<VINP_CANMsgValidEPM2_flg>"
  [CANvalid_bus] -> Bus Selector37/1
  [BHAL_outputs] -> Bus Selector38/1
  Bus Selector38/1 -> TimeToLaneCrossingLeft/1(signal_in)  "<VHAL_TLCL_s>"
  Data Store Read19/1 -> TimeToLaneCrossingLeft/3(Active_fault_flg)
  Bus Selector39/1 -> TimeToLaneCrossingRight/2(msg_valid)  "<VINP_CANMsgValidEPM4_flg>"
  [CANvalid_bus] -> Bus Selector39/1
  [BHAL_outputs] -> Bus Selector40/1
  Bus Selector40/1 -> TimeToLaneCrossingRight/1(signal_in)  "<VHAL_TLCR_s>"
  Data Store Read20/1 -> TimeToLaneCrossingRight/3(Active_fault_flg)
  Bus Selector41/1 -> LaneCrossingLeft/2(msg_valid)  "<VINP_CANMsgValidEPM2_flg>"
  [CANvalid_bus] -> Bus Selector41/1
  [BHAL_outputs] -> Bus Selector42/1
  Bus Selector42/1 -> LaneCrossingLeft/1(signal_in)  "<VHAL_LaneCrosL_flg>"
  Data Store Read21/1 -> LaneCrossingLeft/3(Active_fault_flg)
  Bus Selector43/1 -> LaneCrossingRight/2(msg_valid)  "<VINP_CANMsgValidEPM4_flg>"
  [CANvalid_bus] -> Bus Selector43/1
  [BHAL_outputs] -> Bus Selector44/1
  Bus Selector44/1 -> LaneCrossingRight/1(signal_in)  "<VHAL_LaneCrosR_flg>"
  Data Store Read22/1 -> LaneCrossingRight/3(Active_fault_flg)
  LaneTypeLeft/1(signal_out) -> inputs_bus2/21  "VINP_LaneTypeL_enum_InHAL"
  LaneTypeLeft/2(signal_valid_out) -> inputs_bus2/22  "VINP_LaneTypeLValid_flg_InHAL"
  LaneTypeRight/1(signal_out) -> inputs_bus2/23  "VINP_LaneTypeR_enum_InHAL"
  LaneTypeRight/2(signal_valid_out) -> inputs_bus2/24  "VINP_LaneTypeRValid_flg_InHAL"
  LaneMarkColorLeft/1(signal_out) -> inputs_bus2/25  "VINP_LaneMrkClrL_enum_InHAL"
  LaneMarkColorLeft/2(signal_valid_out) -> inputs_bus2/26  "VINP_LaneMrkClrLValid_flg_InHAL"
  LaneMarkColorRight/1(signal_out) -> inputs_bus2/27  "VINP_LaneMrkClrR_enum_InHAL"
  LaneMarkColorRight/2(signal_valid_out) -> inputs_bus2/28  "VINP_LaneMrkClrRValid_flg_InHAL"
  LaneMarkWidthLeft/1(signal_out) -> inputs_bus2/29  "VINP_LaneMrkWidL_m_InHAL"
  LaneMarkWidthLeft/2(signal_valid_out) -> inputs_bus2/30  "VINP_LaneMrkWidLValid_flg_InHAL"
  LaneMarkWidthRight/1(signal_out) -> inputs_bus2/31  "VINP_LaneMrkWidR_m_InHAL"
  LaneMarkWidthRight/2(signal_valid_out) -> inputs_bus2/32  "VINP_LaneMrkWidRValid_flg_InHAL"
  ViewRangeStartL/1(signal_out) -> inputs_bus2/33  "VINP_ViewRangeStartL_m_InHAL"
  ViewRangeStartL/2(signal_valid_out) -> inputs_bus2/34  "VINP_ViewRangeStartLValid_flg_InHAL"
  ViewRangeStartR/1(signal_out) -> inputs_bus2/35  "VINP_ViewRangeStartR_m_InHAL"
  ViewRangeStartR/2(signal_valid_out) -> inputs_bus2/36  "VINP_ViewRangeStartRValid_flg_InHAL"
  TimeToLaneCrossingLeft/1(signal_out) -> inputs_bus2/41  "VINP_TLCL_s_InHAL"
  TimeToLaneCrossingLeft/2(signal_valid_out) -> inputs_bus2/42  "VINP_TLCLValid_flg_InHAL"
  TimeToLaneCrossingRight/1(signal_out) -> inputs_bus2/43  "VINP_TLCR_s_InHAL"
  TimeToLaneCrossingRight/2(signal_valid_out) -> inputs_bus2/44  "VINP_TLCRValid_flg_InHAL"
  LaneCrossingLeft/1(signal_out) -> inputs_bus2/45  "VINP_LaneCrosL_flg_InHAL"
  LaneCrossingLeft/2(signal_valid_out) -> inputs_bus2/46  "VINP_LaneCrosLValid_flg_InHAL"
  LaneCrossingRight/1(signal_out) -> inputs_bus2/47  "VINP_LaneCrosR_flg_InHAL"
  LaneCrossingRight/2(signal_valid_out) -> inputs_bus2/48  "VINP_LaneCrosRValid_flg_InHAL"
  Bus Selector45/1 -> Terminator7/1  "<VINP_CANMsgValidEPM5_flg>"
  [CANvalid_bus] -> Bus Selector45/1
  Data Store Read23/1 -> Terminator8/1
  Bus Selector48/1 -> Data Type Conversion1/1  "<VHAL_ViewRangeEndL_m>"
  [BHAL_outputs] -> Bus Selector48/1
  Data Type Conversion1/1 -> inputs_bus2/37  "VINP_ViewRangeEndL_m_InHAL"
  Constant1/1 -> inputs_bus2/38  "VINP_ViewRangeEndLValid_flg_InHAL"
  Bus Selector46/1 -> Terminator2/1  "<VINP_CANMsgValidEPM2_flg>"
  [CANvalid_bus] -> Bus Selector46/1
  Data Store Read26/1 -> Terminator3/1
  Bus Selector50/1 -> Data Type Conversion2/1  "<VHAL_ViewRangeEndR_m>"
  [BHAL_outputs] -> Bus Selector50/1
  Data Type Conversion2/1 -> inputs_bus2/39  "VINP_ViewRangeEndR_m_InHAL"
  Constant2/1 -> inputs_bus2/40  "VINP_ViewRangeEndRValid_flg_InHAL"
  Bus Selector49/1 -> Terminator1/1  "<VINP_CANMsgValidEPM4_flg>"
  [CANvalid_bus] -> Bus Selector49/1
  Data Store Read25/1 -> Terminator4/1
  Bus Selector52/1 -> Data Type Conversion3/1  "<VHAL_CamNofObjects_num>"
  [BHAL_outputs] -> Bus Selector52/1
  Data Type Conversion3/1 -> inputs_bus2/49  "VINP_CamNofObjects_num_InHAL"
  Constant3/1 -> inputs_bus2/50  "VINP_CamNofObjectsValid_flg_InHAL"
  Bus Selector47/1 -> Terminator5/1  "<VINP_CANMsgValidEPM5_flg>"
  [CANvalid_bus] -> Bus Selector47/1
  Data Store Read24/1 -> Terminator6/1
  Bus Selector51/1 -> Data Type Conversion4/1  "<VHAL_CamGoDecision_enum>"
  [BHAL_outputs] -> Bus Selector51/1
  Data Type Conversion4/1 -> inputs_bus2/51  "VINP_CamGoDecision_enum_InHAL"
  Constant4/1 -> inputs_bus2/52  "VINP_CamGoDecisionValid_flg_InHAL"
  Bus Selector53/1 -> Terminator9/1  "<VINP_CANMsgValidEPM5_flg>"
  [CANvalid_bus] -> Bus Selector53/1
  Data Store Read27/1 -> Terminator10/1
  Bus Selector54/1 -> Data Type Conversion5/1  "<VHAL_CloseCarDetected_flg>"
  [BHAL_outputs] -> Bus Selector54/1
  Data Type Conversion5/1 -> inputs_bus2/53  "VINP_CloseCarDetected_flg_InHAL"
  Constant5/1 -> inputs_bus2/54  "VINP_CloseCarDetectedValid_flg_InHAL"
  Bus Selector55/1 -> Terminator12/1  "<VINP_CANMsgValidEPM5_flg>"
  [CANvalid_bus] -> Bus Selector55/1
  Data Store Read28/1 -> Terminator11/1
  Bus Selector56/1 -> Data Type Conversion6/1  "<VHAL_RightCarCutIn_flg>"
  [BHAL_outputs] -> Bus Selector56/1
  Data Type Conversion6/1 -> inputs_bus2/55  "VINP_RightCarCutIn_flg_InHAL"
  Constant6/1 -> inputs_bus2/56  "VINP_RightCarCutInValid_flg_InHAL"
  Bus Selector57/1 -> Terminator14/1  "<VINP_CANMsgValidEPM5_flg>"
  [CANvalid_bus] -> Bus Selector57/1
  Data Store Read29/1 -> Terminator13/1
  Bus Selector58/1 -> Data Type Conversion7/1  "<VHAL_LeftCarCutIn_flg>"
  [BHAL_outputs] -> Bus Selector58/1
  Data Type Conversion7/1 -> inputs_bus2/57  "VINP_LeftCarCutIn_flg_InHAL"
  Constant7/1 -> inputs_bus2/58  "VINP_LeftCarCutInValid_flg_InHAL"
  Bus Selector59/1 -> Terminator16/1  "<VINP_CANMsgValidEPM5_flg>"
  [CANvalid_bus] -> Bus Selector59/1
  Data Store Read30/1 -> Terminator15/1
  Bus Selector60/1 -> Data Type Conversion8/1  "<VHAL_CamFSImpairedVD_flg>"
  [BHAL_outputs] -> Bus Selector60/1
  Data Type Conversion8/1 -> inputs_bus2/59  "VINP_CamFSImpairedVD_flg_InHAL"
  Constant8/1 -> inputs_bus2/60  "VINP_CamFSImpairedVDValid_flg_InHAL"
  Bus Selector61/1 -> Terminator18/1  "<VINP_CANMsgValidEPM5_flg>"
  [CANvalid_bus] -> Bus Selector61/1
  Data Store Read31/1 -> Terminator17/1
  Bus Selector62/1 -> Data Type Conversion9/1  "<VHAL_CamFSLowSun_flg>"
  [BHAL_outputs] -> Bus Selector62/1
  Data Type Conversion9/1 -> inputs_bus2/61  "VINP_CamFSLowSun_flg_InHAL"
  Constant9/1 -> inputs_bus2/62  "VINP_CamFSLowSunValid_flg_InHAL"
  Bus Selector63/1 -> Terminator20/1  "<VINP_CANMsgValidEPM5_flg>"
  [CANvalid_bus] -> Bus Selector63/1
  Data Store Read32/1 -> Terminator19/1
  Bus Selector64/1 -> Data Type Conversion10/1  "<VHAL_CamFSBlurImage_flg>"
  [BHAL_outputs] -> Bus Selector64/1
  Data Type Conversion10/1 -> inputs_bus2/63  "VINP_CamFSBlurImage_flg_InHAL"
  Constant10/1 -> inputs_bus2/64  "VINP_CamFSBlurImageValid_flg_InHAL"
  Bus Selector65/1 -> Terminator22/1  "<VINP_CANMsgValidEPM5_flg>"
  [CANvalid_bus] -> Bus Selector65/1
  Data Store Read33/1 -> Terminator21/1
  Bus Selector66/1 -> Data Type Conversion11/1  "<VHAL_CamFSSmearImage_flg>"
  [BHAL_outputs] -> Bus Selector66/1
  Data Type Conversion11/1 -> inputs_bus2/65  "VINP_CamFSSmearImage_flg_InHAL"
  Constant11/1 -> inputs_bus2/66  "VINP_CamFSSmearImageValid_flg_InHAL"
  Bus Selector67/1 -> Terminator24/1  "<VINP_CANMsgValidEPM5_flg>"
  [CANvalid_bus] -> Bus Selector67/1
  Data Store Read34/1 -> Terminator23/1
  Bus Selector68/1 -> Data Type Conversion12/1  "<VHAL_CamFSPartialBlockage_flg>"
  [BHAL_outputs] -> Bus Selector68/1
  Data Type Conversion12/1 -> inputs_bus2/67  "VINP_CamFSPartialBlockage_flg_InHAL"
  Constant12/1 -> inputs_bus2/68  "VINP_CamFSPartialBlockageValid_flg_InHAL"
  Bus Selector69/1 -> Terminator26/1  "<VINP_CANMsgValidEPM5_flg>"
  [CANvalid_bus] -> Bus Selector69/1
  Data Store Read35/1 -> Terminator25/1
  Bus Selector70/1 -> Data Type Conversion13/1  "<VHAL_CamFSFullBlockage_flg>"
  [BHAL_outputs] -> Bus Selector70/1
  Data Type Conversion13/1 -> inputs_bus2/69  "VINP_CamFSFullBlockage_flg_InHAL"
  Constant13/1 -> inputs_bus2/70  "VINP_CamFSFullBlockageValid_flg_InHAL"
  Bus Selector71/1 -> Terminator28/1  "<VINP_CANMsgValidEPM5_flg>"
  [CANvalid_bus] -> Bus Selector71/1
  Data Store Read36/1 -> Terminator27/1
  Bus Selector72/1 -> Data Type Conversion14/1  "<VHAL_CamFSSplashes_flg>"
  [BHAL_outputs] -> Bus Selector72/1
  Data Type Conversion14/1 -> inputs_bus2/71  "VINP_CamFSSplashes_flg_InHAL"
  Constant14/1 -> inputs_bus2/72  "VINP_CamFSSplashesValid_flg_InHAL"
  Bus Selector73/1 -> Terminator30/1  "<VINP_CANMsgValidEPM5_flg>"
  [CANvalid_bus] -> Bus Selector73/1
  Data Store Read37/1 -> Terminator29/1
  Bus Selector74/1 -> Data Type Conversion15/1  "<VHAL_CamFSOutofCal_flg>"
  [BHAL_outputs] -> Bus Selector74/1
  Data Type Conversion15/1 -> inputs_bus2/73  "VINP_CamFSOutofCal_flg_InHAL"
  Constant15/1 -> inputs_bus2/74  "VINP_CamFSOutofCalValid_flg_InHAL"
  Bus Selector75/1 -> Terminator32/1  "<VINP_CANMsgValidEPM5_flg>"
  [CANvalid_bus] -> Bus Selector75/1
  Data Store Read38/1 -> Terminator31/1
  Bus Selector76/1 -> Data Type Conversion16/1  "<VHAL_CamFSCalMisalign_flg>"
  [BHAL_outputs] -> Bus Selector76/1
  Data Type Conversion16/1 -> inputs_bus2/75  "VINP_CamFSCalMisalign_flg_InHAL"
  Constant16/1 -> inputs_bus2/76  "VINP_CamFSCalMisalignValid_flg_InHAL"
  Bus Selector77/1 -> Terminator34/1  "<VINP_CANMsgValidEPM5_flg>"
  [CANvalid_bus] -> Bus Selector77/1
  Data Store Read39/1 -> Terminator33/1
  Bus Selector78/1 -> Data Type Conversion17/1  "<VHAL_CamFSSmearedspots_flg>"
  [BHAL_outputs] -> Bus Selector78/1
  Data Type Conversion17/1 -> inputs_bus2/77  "VINP_CamFSSmearedspots_flg_InHAL"
  Constant17/1 -> inputs_bus2/78  "VINP_CamFSSmearedspotsValid_flg_InHAL"
  Bus Selector79/1 -> Terminator36/1  "<VINP_CANMsgValidEPM5_flg>"
  [CANvalid_bus] -> Bus Selector79/1
  Data Store Read40/1 -> Terminator35/1
  Bus Selector80/1 -> Data Type Conversion18/1  "<VHAL_CamFSSpotHalosFrozen_flg>"
  [BHAL_outputs] -> Bus Selector80/1
  Data Type Conversion18/1 -> inputs_bus2/79  "VINP_CamFSSpotHalosFrozen_flg_InHAL"
  Constant18/1 -> inputs_bus2/80  "VINP_CamFSSpotHalosFrozenValid_flg_InHAL"
  Bus Selector81/1 -> Terminator38/1  "<VINP_CANMsgValidEPM5_flg>"
  [CANvalid_bus] -> Bus Selector81/1
  Data Store Read41/1 -> Terminator37/1
  Bus Selector82/1 -> Data Type Conversion19/1  "<VHAL_CamFSFoggySpots_flg>"
  [BHAL_outputs] -> Bus Selector82/1
  Data Type Conversion19/1 -> inputs_bus2/81  "VINP_CamFSFoggySpots_flg_InHAL"
  Constant19/1 -> inputs_bus2/82  "VINP_CamFSFoggySpotsValid_flg_InHAL"
  Bus Selector83/1 -> Terminator40/1  "<VINP_CANMsgValidEPM5_flg>"
  [CANvalid_bus] -> Bus Selector83/1
  Data Store Read42/1 -> Terminator39/1
  Bus Selector84/1 -> Data Type Conversion20/1  "<VHAL_CamFSSunRay_flg>"
  [BHAL_outputs] -> Bus Selector84/1
  Data Type Conversion20/1 -> inputs_bus2/83  "VINP_CamFSSunRay_flg_InHAL"
  Constant20/1 -> inputs_bus2/84  "VINP_CamFSSunRayValid_flg_InHAL"
  Gain/1 -> LaneC0Left/1(signal_in)
  Gain1/1 -> LaneC1Left/1(signal_in)
  Gain2/1 -> LaneC2Left/1(signal_in)
  Gain3/1 -> LaneC3Left/1(signal_in)
  Gain4/1 -> LaneC0Right/1(signal_in)
  Gain5/1 -> LaneC1Right/1(signal_in)
  Gain6/1 -> LaneC2Right/1(signal_in)
  Gain7/1 -> LaneC3Right/1(signal_in)
  [BHAL_outputs] -> Cam_Obstacle_1/1(BHAL_outputs)
  [CANvalid_bus] -> Cam_Obstacle_1/2(CANvalid_bus)
  [BHAL_outputs] -> Cam_Obstacle_2/1(BHAL_outputs)
  [CANvalid_bus] -> Cam_Obstacle_2/2(CANvalid_bus)
  Cam_Obstacle_1/1(Cam_Obstacle_1) -> inputs_bus2/87
  Cam_Obstacle_2/1(Cam_Obstacle_2) -> inputs_bus2/88
  [BHAL_outputs] -> Cam_Obstacle_3/1(BHAL_outputs)
  [CANvalid_bus] -> Cam_Obstacle_3/2(CANvalid_bus)
  Cam_Obstacle_3/1(Cam_Obstacle_3) -> inputs_bus2/89
  Cam_Obstacle_4/1(Cam_Obstacle_4) -> inputs_bus2/90
  [BHAL_outputs] -> Cam_Obstacle_4/1(BHAL_outputs)
  [CANvalid_bus] -> Cam_Obstacle_4/2(CANvalid_bus)
  Cam_Obstacle_5/1(Cam_Obstacle_5) -> inputs_bus2/91
  [BHAL_outputs] -> Cam_Obstacle_5/1(BHAL_outputs)
  [CANvalid_bus] -> Cam_Obstacle_5/2(CANvalid_bus)
  Cam_Obstacle_6/1(Cam_Obstacle_6) -> inputs_bus2/92
  [BHAL_outputs] -> Cam_Obstacle_6/1(BHAL_outputs)
  [CANvalid_bus] -> Cam_Obstacle_6/2(CANvalid_bus)
  Cam_Obstacle_7/1(Cam_Obstacle_7) -> inputs_bus2/93
  [BHAL_outputs] -> Cam_Obstacle_7/1(BHAL_outputs)
  [CANvalid_bus] -> Cam_Obstacle_7/2(CANvalid_bus)
  Cam_Obstacle_8/1(Cam_Obstacle_8) -> inputs_bus2/94
  [BHAL_outputs] -> Cam_Obstacle_8/1(BHAL_outputs)
  [CANvalid_bus] -> Cam_Obstacle_8/2(CANvalid_bus)
  Cam_Obstacle_9/1(Cam_Obstacle_9) -> inputs_bus2/95
  [BHAL_outputs] -> Cam_Obstacle_9/1(BHAL_outputs)
  [CANvalid_bus] -> Cam_Obstacle_9/2(CANvalid_bus)
  Cam_Obstacle_10/1(Cam_Obstacle_10) -> inputs_bus2/96
  [BHAL_outputs] -> Cam_Obstacle_10/1(BHAL_outputs)
  [CANvalid_bus] -> Cam_Obstacle_10/2(CANvalid_bus)
  [BHAL_outputs] -> Cam_Left_Next_Lane/1(BHAL_outputs)
  [CANvalid_bus] -> Cam_Left_Next_Lane/2(CANvalid_bus)
  Cam_Left_Next_Lane/1(VINP_LeftNextLane_BUS_InHAL) -> inputs_bus2/85
  [BHAL_outputs] -> Cam_Right_Next_Lane/1(BHAL_outputs)
  [CANvalid_bus] -> Cam_Right_Next_Lane/2(CANvalid_bus)
  Cam_Right_Next_Lane/1(VINP_RightNextLane_BUS_InHAL) -> inputs_bus2/86
